simulink model "TosAeb"  (R2021a)
settings: Solver=FixedStepDiscrete  StartTime=0.0  StopTime=inf  FixedStep=0.01  RelTol=1e-3
undefined symbols (not defined in the file or in its folder): ACCModSts, AccSts1, AEB_ObjectMovingOrientation, AEB_ObjectMovingState, CN_BTCActive, CN_CurrentGearR, CN_CurrentGearR_DHT, CN_PTCActive, CN_VDCActive, CN_VSC_EspOff, CN_VSC_FCW_AEB_Error, CN_VSC_FCW_AEB_NoDispay, CN_VSC_FCW_AEB_PerfmDgdtn, CN_VSC_FCW_AEB_TempUnAvlbl, CN_VSC_VehStandstill, CNaccelAvoidMax, CNaccelAvoidMin, CNbrakePedalPress, CNcomfortStrLeftAccelMax, CNcomfortStrLeftAccelMin, CNcomfortStrRightAccelMax, CNcomfortStrRightAccelMin, CNDrvDoorOpen, CNDrvModeEco, CNDrvModeNorm, CNDrvModeOther, CNDrvModeSport, CNDrvSBRreminder, CNNull, CNobjInvalidID, CNPassDoorOpen, CNPassSBRreminder, CNPEBSAccelNegInf, CNPEBSAccelNull, CNPEBSTimeInf, CNPEBSTimeNull, CNSTOPMAX, CNSTOPNULL, CNsubjWidth, CNSwtOFF, CNSwtON, CNTTCMAX, CNTTCNULL, CNVehConfigAEBCycNone, CNVehConfigAEBNone, CNVehConfigAEBPedNone, CNVehConfigFCWNone, CNVehConfigJANone, CollisionSeverity, FALSE, FCWSnvty, JACycObjCalcInfoDefaultParams, JAPedObjCalcInfoDefaultParams, JAVehObjCalcInfoDefaultParams, latCycObjCalcInfoDefaultParams, latPedObjCalcInfoDefaultParams, longObjCalcInfoDefaultParams, ModSts, movingStatus, ObjectType ... (+1112)
libraries: simulink x3031, AEBSFunctionLib x50, Ta_Library x33, AEBSStateMachineLib x23, Mylibrary x12, simulink_extras x7, ObjSelect_lib x3, dspstat3 x2
(part 100 of 102)

== stateflow chart TosAeb/FcwAebJa_Step_sys/ALG_AEBSystem/ALG_AEBController/AEB_LatObjInfoCalcApp/LatCycObjCalc/LatCycObjCalc/latCycObjInfoCalc/ObjColiisionRiskProbCalc/CollisionRiskProbCalc/ObjReginCorrCalc/ObjReginCorrCalc/Chart ==
  note: ObjUp2Dwn
  note: ObjDwn2Up
  note: ObjLeft2Right
  note: ObjRight2Left
  (default) -> (junction 2)
  (junction 2) -> (junction 3) [order 1] : [vxAbsObj < 0]
  (junction 2) -> (junction 26) [order 2]
  (junction 3) -> (junction 5) : {
      | 	tObjStop = abs(vxAbsObj/ax_Max);
      | }
  (junction 26) -> (junction 28) [order 1] : [vxAbsObj > 0]
  (junction 26) -> (junction 30) [order 2] : {
      | 	temp_tPredTimeMax_X = 0;
      | 	temp_tPredTimeMin_X = 0;
      | }
  (junction 28) -> (junction 15) : {
      | 	tObjStop = abs(vxAbsObj/ax_Min);
      | }
  (junction 5) -> (junction 7) [order 1] : [tObjStop < tPredTime]
  (junction 5) -> (junction 11) [order 2] : {
      | 	temp_tPredTimeMax_X = tPredTime;
      | 	temp_tPredTimeMin_X = tPredTime;
      | }
  (junction 7) -> (junction 9) : {
      | 	temp_tPredTimeMax_X = tObjStop;
      | 	temp_tPredTimeMin_X = tPredTime;
      | }
  (junction 15) -> (junction 19) [order 1] : [tObjStop < tPredTime]
  (junction 15) -> (junction 23) [order 2] : {
      | 	temp_tPredTimeMax_X = tPredTime;
      | 	temp_tPredTimeMin_X = tPredTime;
      | }
  (junction 19) -> (junction 21) : {
      | 	temp_tPredTimeMax_X = tPredTime;
      | 	temp_tPredTimeMin_X = tObjStop;
      | }
  (junction 23) -> (junction 30)
  (junction 21) -> (junction 23)
  (junction 11) -> (junction 21)
  (junction 9) -> (junction 11)
  (junction 30) -> (junction 53)
  (junction 53) -> (junction 65) [order 1] : [vyAbsObj < 0]
  (junction 53) -> (junction 36) [order 2]
  (junction 65) -> (junction 64) : {
      | 	tObjStop = abs(vyAbsObj/ay_Max);
      | }
  (junction 36) -> (junction 40) [order 1] : [vyAbsObj > 0]
  (junction 36) -> (junction 35) [order 2] : {
      | 	temp_tPredTimeMax_Y= 0;
      | 	temp_tPredTimeMin_Y= 0;
      | }
  (junction 40) -> (junction 50) : {
      | 	tObjStop = abs(vyAbsObj/ay_Min);
      | }
  (junction 64) -> (junction 56) [order 1] : [tObjStop < tPredTime]
  (junction 64) -> (junction 51) [order 2] : {
      | 	temp_tPredTimeMax_Y = tPredTime;
      | 	temp_tPredTimeMin_Y= tPredTime;
      | }
  (junction 56) -> (junction 42) : {
      | 	temp_tPredTimeMax_Y = tObjStop;
      | 	temp_tPredTimeMin_Y = tPredTime;
      | }
  (junction 50) -> (junction 54) [order 1] : [tObjStop < tPredTime]
  (junction 50) -> (junction 58) [order 2] : {
      | 	temp_tPredTimeMax_Y = tPredTime;
      | 	temp_tPredTimeMin_Y= tPredTime;
      | }
  (junction 54) -> (junction 39) : {
      | 	temp_tPredTimeMax_Y = tPredTime;
      | 	temp_tPredTimeMin_Y = tObjStop;
      | }
  (junction 58) -> (junction 35)
  (junction 39) -> (junction 58)
  (junction 51) -> (junction 39)
  (junction 42) -> (junction 51)
  (junction 35) -> (junction 67) : {
      | 	tPredTimeMax_X = temp_tPredTimeMax_X;
      | 	tPredTimeMin_X = temp_tPredTimeMin_X;
      | 	tPredTimeMax_Y = temp_tPredTimeMax_Y;
      | 	tPredTimeMin_Y = temp_tPredTimeMin_Y;
      | }
  data: ax_Max input; ax_Min input; ay_Max input; ay_Min input; tPredTime input; vxAbsObj input; vyAbsObj input; tPredTimeMax_X output single; tPredTimeMin_X output single; tPredTimeMax_Y output single; tPredTimeMin_Y output single; tObjStop local single; temp_tPredTimeMax_X local single; temp_tPredTimeMax_Y local single; temp_tPredTimeMin_X local single; temp_tPredTimeMin_Y local single

== stateflow chart TosAeb/FcwAebJa_Step_sys/ALG_AEBSystem/ALG_AEBController/AEB_LongTgtInfoCalc/LongObjCalcInfoCalc/ObjAbsInfoTrans/ObjFusQualityCal ==
  state [deltaDxThres,deltaDyThres,deltaFC_FRVxThres,deltaFR_FusVxThres,deltaFC_FusVxThres] = SensorThres(subjVel) [function]
  (default) -> (junction 9)
  (junction 9) -> (junction 11) [order 1] : [ObjectID ~= CNobjInvalidID &&...
      | ObjectTypeCameraConfirmed == true]
  (junction 9) -> (junction 27) [order 2] : {
      |    ObjFusQuality = false;
      | }
  (junction 11) -> (junction 13)
  (junction 13) -> (junction 15) [order 1] : [FR_ObjectID ~= CNobjInvalidID &&...
      | FC_ObjectID ~= CNobjInvalidID]
  (junction 13) -> (junction 24) [order 2] : {
      |    ObjFusQuality = false;
      | }
  (junction 15) -> (junction 17) : {
      |         temp_Dx = abs(FR_Dx - FC_Dx);
      |         temp_Dy = abs(FR_Dy - FC_Dy);
      |         temp_FC_FR_Vx = abs(FC_Vx - FR_Vx);
      |         temp_FC_Fus_Vx = abs(FC_Vx - Fus_Vx);
      |         temp_FR_Fus_Vx = abs(FR_Vx - Fus_Vx);
      |         [deltaDxThres,...
      |          deltaDyThres,...
      |          deltaFC_FRVxThres,...
      |          deltaFR_FusVxThres,...
      |          deltaFC_FusVxThres] = SensorThres(subjVel);
      | }
  (junction 17) -> (junction 19) [order 1] : [temp_Dx > deltaDxThres ||...
      | temp_Dy > deltaDyThres]
  (junction 17) -> (junction 59) [order 2]
  (junction 19) -> (junction 21) : {
      |    ObjFusQuality = false;
      | }
  (junction 59) -> (junction 66) [order 1] : [Fus_ObjectType ==ObjectTypeCopy.CAR ||...
      | Fus_ObjectType ==ObjectTypeCopy.TRUCK]
  (junction 59) -> (junction 33) [order 2] : {
      |    ObjFusQuality = true;
      | }
  (junction 66) -> (junction 68)
  (junction 68) -> (junction 61) [order 1] : [(temp_FC_FR_Vx>deltaFC_FRVxThres&&...
      | temp_FR_Fus_Vx<deltaFR_FusVxThres)||...
      | (temp_FC_FR_Vx<deltaFC_FRVxThres&&...
      | temp_FR_Fus_Vx>deltaFR_FusVxThres)]
  (junction 68) -> (junction 70) [order 2] : {
      |    ObjFusQuality = true;
      | }
  (junction 61) -> (junction 63) : {
      |    ObjFusQuality = false;
      | }
  (junction 24) -> (junction 27)
  (junction 33) -> (junction 24)
  (junction 70) -> (junction 33)
  (junction 63) -> (junction 70)
  (junction 21) -> (junction 63)
  (junction 27) -> (junction 30)
  data: subjVel function_input; deltaDxThres function_output single; deltaDyThres function_output single; deltaFC_FRVxThres function_output; deltaFR_FusVxThres function_output; deltaFC_FusVxThres function_output; ObjectID input size=1; ObjectTypeCameraConfirmed input size=1; Fus_ObjectType input Enum: ObjectTypeCopy; FC_ObjectID input size=1; FR_ObjectID input size=1; FR_Dx input size=1; FR_Dy input size=1; FR_Vx input; FC_Dx input size=1; FC_Dy input size=1; CNobjInvalidID parameter size=1; ObjFusQuality output size=1; temp_Dx local single size=1; temp_Dy local single size=1; deltaDyThres local single size=1 =single(0); deltaDxThres local single size=1 =single(0); temp_FC_Fus_Vx local single; deltaFC_FRVxThres local single =single(0); deltaFR_FusVxThres local single =single(0); FC_Vx input; Fus_Vx input; subjVel input size=1; temp_FC_FR_Vx local single; temp_FR_Fus_Vx local single; deltaFC_FusVxThres local single =single(0)

== stateflow chart TosAeb/FcwAebJa_Step_sys/ALG_AEBSystem/ALG_AEBController/AEB_LatObjInfoCalcApp/LatCycObjCalc/LatCycObjCalc/latCycObjInfoCalc/ObjColiisionRiskProbCalc/CollisionRiskProbCalcFlow ==
  (default) -> (junction 11)
  (junction 11) -> (junction 2) : {
      | 	tPredictTime = 0;
      | 	temp_collisionRiskProbMax = 0;
      | }
  (junction 2) -> (junction 6) [order 1] : [tPredictTime < tMaxPredTime]
  (junction 41) -> (junction 2)
  (junction 2) -> (junction 13) [order 2] : {
      | 	collisionRiskProbMax = temp_collisionRiskProbMax;
      | 	preCollisonMaxTime = temp_preCollisonMaxTime;
      | 	dxCollisionRiskProbOut = dxCollisionRiskProb;
      | 	dyCollisionRiskProbOut = dyCollisionRiskProb;
      | }
  (junction 6) -> (junction 32) : {
      | 	tPredictTimeOut = tPredictTime;
      | 	CollisionRiskProbCalcFunc;
      | }
  (junction 32) -> (junction 34) [order 1] : [collisionRiskProb > temp_collisionRiskProbMax]
  (junction 32) -> (junction 8) [order 2]
  (junction 34) -> (junction 35) : {
      | 	temp_collisionRiskProbMax = collisionRiskProb;
      | 	temp_preCollisonMaxTime =  tPredictTime;
      | }
  (junction 35) -> (junction 8)
  (junction 8) -> (junction 41) : {
      | 	tPredictTime += stepTime;
      | }
  data: collisionRiskProbMax output single; dxCollisionRiskProbOut output single; dyCollisionRiskProbOut output single; preCollisonMaxTime output single; stepTime input single; tMaxPredTime input single; collisionRiskProb input single; dyCollisionRiskProb input single; tPredictTimeOut output single; tPredictTime local single; temp_collisionRiskProbMax local single; temp_preCollisonMaxTime local single; dxCollisionRiskProb input single
  events: CollisionRiskProbCalcFunc output FUNCTION_CALL_EVENT

== stateflow chart TosAeb/FcwAebJa_Step_sys/ALG_AEBSystem/ALG_AEBController/AEB_LatObjInfoCalcApp/LatCycObjCalc/LatCycObjCalc/latCycObjInfoCalc/ObjCrossFlgCal/CollisionProbCalcFlow ==
  (default) -> (junction 11)
  (junction 11) -> (junction 2) : {
      |   tPredictTime = 0;
      |   TTC = 256;
      | }
  (junction 2) -> (junction 6) [order 1] : [tPredictTime < tMaxPredTime]
  (junction 30) -> (junction 2) [order 2]
  (junction 2) -> (junction 13) [order 2]
  (junction 6) -> (junction 8) : {
      | 	tPredictTimeOut = tPredictTime;
      | 	CollisionProbFunc;
      | }
  (junction 8) -> (junction 30) : {
      | 	tPredictTime += stepTime;
      | }
  (junction 30) -> (junction 22) [order 1] : [preCollisionProb == true]
  (junction 22) -> (junction 13) : {
      |   TTC = tPredictTimeOut;
      | }
  (junction 13) -> (junction 25)
  data: stepTime input single; tMaxPredTime input single; tPredictTimeOut output single; tPredictTime local single; preCollisionProb input; TTC output single
  events: CollisionProbFunc output FUNCTION_CALL_EVENT

== stateflow chart TosAeb/FcwAebJa_Step_sys/ALG_AEBSystem/ALG_AEBController/AEB_LatObjInfoCalcApp/LatCycObjCalc/LatCycObjCalc/latCycObjInfoCalc/ObjCrossFlgCal/CycPreCollisionCalcFunc/ObjLenthWidthCalc/ObjLengthWidthCalc ==
  (default) -> (junction 10) : {
      | 	ObjLengthCorr = P_LatCycCA_PreCollisionCycLengthThres;
      | 	ObjWidthCorr = P_LatCycCA_PreCollisionCycWidthThres;
      | }
  (junction 10) -> (junction 12) [order 1] : [(abs(ObjectYawAngle) > 45 &&...
      | abs(ObjectYawAngle) < 135)||...
      | ObjectMovingOrientation == AEB_ObjectMovingOrientation.Crossing]
  (junction 10) -> (junction 16) [order 2]
  (junction 12) -> (junction 14) : {
      | 	ObjLengthCorr = ObjectWidth;
      | 	ObjWidthCorr = ObjectLength;
      | }
  (junction 14) -> (junction 16)
  (junction 16) -> (junction 19)
  data: ObjectYawAngle input; ObjectMovingOrientation input; ObjectLength input; ObjectWidth input; ObjLengthCorr output single; ObjWidthCorr output single; P_LatCycCA_PreCollisionCycLengthThres input; P_LatCycCA_PreCollisionCycWidthThres input

== stateflow chart TosAeb/FcwAebJa_Step_sys/ALG_AEBSystem/ALG_AEBController/AEB_LatObjInfoCalcApp/LatCycObjCalc/LatCycObjCalc/latCycObjInfoCalc/ObjCrossFlgCal/CycPreCollisionCalcFunc/ObjLenthWidthCalc/ObjLengthWidthCalc1 ==
  (default) -> (junction 32)
  (junction 32) -> (junction 34) [order 1] : [ObjectTypeCameraConfirmed == true]
  (junction 32) -> (junction 30) [order 2] : {
      | 	ObjectLength = P_LatCycCA_PreCollisionCycLengthThres;
      | 	ObjectWidth = P_LatCycCA_PreCollisionCycWidthThres;
      | }
  (junction 34) -> (junction 36) : {
      | 	ObjectLength = ObjectLengthIn;
      | 	ObjectWidth = ObjectWidthIn;
      | }
  (junction 36) -> (junction 30)
  (junction 30) -> (junction 10)
  (junction 10) -> (junction 12) [order 1] : [(abs(ObjectYawAngle) > 45 &&...
      | abs(ObjectYawAngle) < 135)||...
      | ObjectMovingOrientation == AEB_ObjectMovingOrientation.Crossing]
  (junction 10) -> (junction 16) [order 2]
  (junction 12) -> (junction 14) : {
      |       temp = ObjectLength;
      | 	ObjectLength = ObjectWidth;
      | 	ObjectWidth = temp;
      | }
  (junction 14) -> (junction 16)
  (junction 16) -> (junction 19) : {
      |    ObjectLength = min(ObjectLength,2.5);
      |    ObjectWidth = min(ObjectWidth,1.5);
      | }
  data: ObjectTypeCameraConfirmed input boolean; ObjectLengthIn input; ObjectWidthIn input; ObjectYawAngle input; ObjectMovingOrientation input; ObjectLength output single; ObjectWidth output single; P_LatCycCA_PreCollisionCycLengthThres input; P_LatCycCA_PreCollisionCycWidthThres input; temp local single

== stateflow chart TosAeb/FcwAebJa_Step_sys/ALG_AEBSystem/ALG_AEBController/AEB_LatObjInfoCalcApp/LatCycObjCalc/LatCycObjCalc/latCycObjInfoCalc/ObjCrossFlgCal/CycPreCollisionCalcFunc/PreCrossFlgCalcFlow ==
  state pedPreCollisionFlg = VectorCrossAlgorithm(x1,y1,x2,y2,m1,n1,m2,n2) [function]
  (default) -> (junction 59) : {
      | 	pedPreCollisionFlg = false;
      | 	i = 0;
      | }
  (junction 59) -> (junction 58) [order 1] : [ i < 4]
  (junction 58) -> (junction 48) : {
      | 	p = (i+1)%%4;
      | 	j = 0;
      | }
  (junction 48) -> (junction 46) [order 1] : [j < 4]
  (junction 46) -> (junction 49) : {
      | 	q = (j+1)%%4;
      | 	pedPreCollisionFlg = VectorCrossAlgorithm(subjDxPre[i],subjDyPre[i],subjDxPre[p],subjDyPre[p],...
      | 	objDxPre[j],objDyPre[j],objDxPre[q],objDyPre[q]);
      | }
  (junction 60) -> (junction 59) : {
      | 	i++;
      | }
  (junction 59) -> (junction 61) [order 2]
  (junction 49) -> (junction 48) [order 2] : {
      | 	j++;
      | }
  (junction 48) -> (junction 60) [order 2]
  (junction 49) -> (junction 64) [order 1] : [pedPreCollisionFlg == true]
  (junction 64) -> (junction 61)
  (junction 61) -> (junction 67)
  data: x1 function_input single; y1 function_input single; x2 function_input single; y2 function_input single; m1 function_input single; n1 function_input single; m2 function_input single; n2 function_input single; pedPreCollisionFlg function_output; subjDxPre input single; subjDyPre input single; objDxPre input single; objDyPre input single; j local single; i local single; p local single; q local single; pedPreCollisionFlg output boolean

== stateflow chart TosAeb/FcwAebJa_Step_sys/ALG_AEBSystem/ALG_AEBController/AEB_LatObjInfoCalcApp/LatCycObjCalc/TargetSelectCalc/tgtObjFlow1 ==
  state TargetSelectFunc [function]
    (default) -> (junction 63)
    (junction 63) -> (junction 75) [order 1] : [ObjPreSelectCondFufilled(index) == false]
    (junction 63) -> (junction 94) [order 2] : {temp = latCycCalcInfo(index).CollisionRiskProb;}
    (junction 75) -> (junction 72)
    (junction 94) -> (junction 93) [order 1] : %[latCycCalcInfo(index).CollisionRiskProb > 0.55]
        | [temp > P_LatCycCA_SelectObjCollisionRiskProbThres]
    (junction 94) -> (junction 187) [order 2]
    (junction 93) -> (junction 225) : {
        | 	tgtIsFind = true;
        | }
    (junction 225) -> (junction 227) [order 1] : [ObjPreSelectCondFufilled(tgtIndex) == false]
    (junction 225) -> (junction 185) [order 2]
    (junction 227) -> (junction 229) : {
        | 	tgtIndex = index;
        | }
    (junction 185) -> (junction 143) [order 1] : [latCycCalcInfo(index).CollisionRiskProb > ...
        | latCycCalcInfo(tgtIndex).CollisionRiskProb]
    (junction 185) -> (junction 149) [order 2]
    (junction 143) -> (junction 145) : {
        | 	tgtIndex = index;
        | }
    (junction 149) -> (junction 151) [order 1] : [abs(latCycCalcInfo(index).CollisionRiskProb) == ...
        | abs(latCycCalcInfo(tgtIndex).CollisionRiskProb)]
    (junction 149) -> (junction 69) [order 2]
    (junction 151) -> (junction 157) : {
        | 	temp1=abs(latCycCalcInfo(index).latCycObjRawInfo.dy);
        | 	temp2 = abs(latCycCalcInfo(tgtIndex).latCycObjRawInfo.dy);
        | }
    (junction 157) -> (junction 158) [order 1] : [temp1 <= temp2]
    (junction 157) -> (junction 153) [order 2]
    (junction 158) -> (junction 160) : {
        | 	tgtIndex = index;
        | }
    (junction 229) -> (junction 145)
    (junction 72) -> (junction 229)
    (junction 145) -> (junction 160)
    (junction 69) -> (junction 187)
    (junction 153) -> (junction 69)
    (junction 160) -> (junction 153)
    (junction 187) -> (junction 81)
  state TgtIndexSearch [function]
    (default) -> (junction 233)
    (junction 233) -> (junction 234) : {
        |     index = 1;
        | }
    (junction 234) -> (junction 235) [order 1] : [index <= 10]
    (junction 238) -> (junction 234)
    (junction 234) -> (junction 239) [order 2]
    (junction 235) -> (junction 236)
    (junction 236) -> (junction 237) [order 1] : [latCycCalcInfo(index).latCycObjRawInfo.ObjectID == tgtIndexK1]
    (junction 236) -> (junction 238) [order 2] : {
        |    index = index+1;
        | }
    (junction 237) -> (junction 240) : {
        |    tgtIndexK1 = index;
        | }
    (junction 240) -> (junction 239)
    (junction 239) -> (junction 241)
  (default) -> (junction 191) : {
      | 	tgtIndex = 1;
      | 	tgtIsFind = false;
      |       tgtIndexK1 = uint8(1);
      | }
  (junction 191) -> (junction 198)
  (junction 198) -> (junction 196) [order 1] : [TargetIDK1 ~= 0]
  (junction 198) -> (junction 216) [order 2]
  (junction 196) -> (junction 200) : {
      |    TgtIndexSearch();
      | }
  (junction 200) -> (junction 193) [order 1] : [ObjPreSelectCondFufilled(tgtIndexK1) == true]
  (junction 200) -> (junction 213) [order 2]
  (junction 193) -> (junction 205)
  (junction 213) -> (junction 216)
  (junction 205) -> (junction 213) [order 2]
  (junction 205) -> (junction 207) [order 1] : [latCycCalcInfo(tgtIndexK1).CollisionRiskProb >P_LatCycCA_SelectTgtCollisionRiskProbThres ]
  (junction 216) -> (junction 112) : {
      | 	index = uint8(1);
      | }
  (junction 207) -> (junction 211) : {
      | 	tgtIndex = tgtIndexK1;
      | 	tgtIsFind = true;
      | }
  (junction 112) -> (junction 110) [order 1] : [index <= 10]
  (junction 169) -> (junction 112)
  (junction 112) -> (junction 209) [order 2]
  (junction 110) -> (junction 113) : {
      | 	TargetSelectFunc();
      | }
  (junction 113) -> (junction 169) : {
      | 	index = index + 1;
      | }
  (junction 211) -> (junction 209)
  (junction 209) -> (junction 114)
  (junction 114) -> (junction 172) [order 1] : [tgtIsFind == true]
  (junction 114) -> (junction 176) [order 2] : {
      | 	tgtObj = latCycObjCalcInfoDefaultParams;
      | }
  (junction 172) -> (junction 174) : {
      | 	tgtObj = latCycCalcInfo(tgtIndex);
      | }
  (junction 174) -> (junction 176)
  (junction 176) -> (junction 179)
  data: latCycCalcInfo input Bus: BusLatCycObjCalcInfo size=10; ObjPreSelectCondFufilled input size=10; index local uint8; tgtObj output Bus: BusLatCycObjCalcInfo; temp1 local; temp2 local; tgtIsFind output; latCycObjCalcInfoDefaultParams parameter Bus: BusLatCycObjCalcInfo; tgtIndex output uint8; temp local; P_LatCycCA_SelectObjCollisionRiskProbThres input; P_LatCycCA_SelectTgtCollisionRiskProbThres input; TargetIDK1 input uint16; tgtIndexK1 local uint8

== stateflow chart TosAeb/FcwAebJa_Step_sys/ALG_AEBSystem/ALG_AEBController/AEB_LatObjInfoCalcApp/LatPedObjCalc/LatPedCollisonAssessInfo/LatPedCollisonAssessInfo/LatPedCollisionSeverityCalc/LatCollsionSeverityJudgeFlow ==
  note: DistanceTriggerForPrefill == true
  note: 变更1和2的条件
  (default) -> (junction 88)
  (junction 88) -> (junction 89) [order 1] : [ latCollsionSeverityK1== CollisionSeverity.None]
  (junction 88) -> (junction 92) [order 2]
  (junction 89) -> (junction 90) : {
      | 	PseverityLowThres = ...
      | P_LatPedCA_Lvl1SeverityThresCurrentLvl0;
      | 	PseverityMediumThres = ...
      | P_LatPedCA_Lvl2SeverityThresCurrentLvl0;
      | 	PseverityHighThres = ...
      | P_LatPedCA_Lvl3SeverityThresCurrentLvl0;
      | }
  (junction 92) -> (junction 93) [order 1] : [ latCollsionSeverityK1== CollisionSeverity.Low]
  (junction 92) -> (junction 96) [order 2]
  (junction 93) -> (junction 94) : {
      | 	PseverityLowThres = ...
      | P_LatPedCA_Lvl1SeverityThresCurrentLvl1;
      | 	PseverityMediumThres = ...
      | P_LatPedCA_Lvl2SeverityThresCurrentLvl1;
      | 	PseverityHighThres = ...
      | P_LatPedCA_Lvl3SeverityThresCurrentLvl1;
      | }
  (junction 96) -> (junction 97) [order 1] : [ latCollsionSeverityK1== CollisionSeverity.Medium]
  (junction 96) -> (junction 100) [order 2] : {
      | 	PseverityLowThres = ...
      | P_LatPedCA_Lvl1SeverityThresCurrentLvl3;
      | 	PseverityMediumThres = ...
      | P_LatPedCA_Lvl2SeverityThresCurrentLvl3;
      | 	PseverityHighThres = ...
      | P_LatPedCA_Lvl3SeverityThresCurrentLvl3;
      | }
  (junction 97) -> (junction 98) : {
      | 	PseverityLowThres = ...
      | P_LatPedCA_Lvl1SeverityThresCurrentLvl2;
      | 	PseverityMediumThres = ...
      | P_LatPedCA_Lvl2SeverityThresCurrentLvl2;
      | 	PseverityHighThres = ...
      | P_LatPedCA_Lvl3SeverityThresCurrentLvl2;
      | }
  (junction 98) -> (junction 100)
  (junction 94) -> (junction 98)
  (junction 90) -> (junction 94)
  (junction 100) -> (junction 106)
  (junction 106) -> (junction 108) [order 1] : [TargetID == CNobjInvalidID]
  (junction 108) -> (junction 144) : {
      | 	tempLatCollsionSeverity = CollisionSeverity.None;
      | }
  (junction 106) -> (junction 124) [order 2]
  (junction 124) -> (junction 120) [order 1] : [ pedColliisonRisk > PseverityHighThres]
  (junction 124) -> (junction 189) [order 2]
  (junction 120) -> (junction 155)
  (junction 155) -> (junction 189) [order 2]
  (junction 155) -> (junction 156) [order 1] : [ longDistCond4CollisionSeverityHigh == true]
  (junction 189) -> (junction 187)
  (junction 156) -> (junction 158) : {
      | 	tempLatCollsionSeverity = ...
      | 	CollisionSeverity.High;
      | }
  (junction 187) -> (junction 113) [order 1] : [ pedColliisonRisk > PseverityMediumThres]
  (junction 187) -> (junction 193) [order 2]
  (junction 113) -> (junction 163)
  (junction 163) -> (junction 193) [order 2]
  (junction 163) -> (junction 164) [order 1] : [longDistCond4CollisionSeverityMedium == true]
  (junction 193) -> (junction 196)
  (junction 164) -> (junction 112) : {
      | 	tempLatCollsionSeverity = ...
      | 	CollisionSeverity.Medium;
      | }
  (junction 196) -> (junction 136) [order 1] : [ pedColliisonRisk > PseverityLowThres]
  (junction 196) -> (junction 198) [order 2]
  (junction 136) -> (junction 171)
  (junction 171) -> (junction 198) [order 2]
  (junction 171) -> (junction 172) [order 1] : [longDistCond4CollisionSeverityLow == true]
  (junction 198) -> (junction 119) : {
      | 	tempLatCollsionSeverity = CollisionSeverity.None;
      | }
  (junction 172) -> (junction 174) : {
      | 	tempLatCollsionSeverity = ...
      | 	CollisionSeverity.Low;
      | }
  (junction 158) -> (junction 112)
  (junction 144) -> (junction 158)
  (junction 112) -> (junction 174)
  (junction 174) -> (junction 119)
  (junction 119) -> (junction 132) : {
      | 	latCollsionSeverity = tempLatCollsionSeverity;
      | }
  data: TargetID input; pedColliisonRisk input single; longDistCond4CollisionSeverityLow input; longDistCond4CollisionSeverityMedium input; longDistCond4CollisionSeverityHigh input; latCollsionSeverity output Enum: CollisionSeverity; CNobjInvalidID parameter; PseverityLowThres local single; PseverityMediumThres local single; PseverityHighThres local single; tempLatCollsionSeverity local Enum: CollisionSeverity; P_LatPedCA_Lvl1SeverityThresCurrentLvl0 input; P_LatPedCA_Lvl2SeverityThresCurrentLvl0 input; P_LatPedCA_Lvl3SeverityThresCurrentLvl0 input; P_LatPedCA_Lvl1SeverityThresCurrentLvl1 input; P_LatPedCA_Lvl2SeverityThresCurrentLvl1 input; P_LatPedCA_Lvl3SeverityThresCurrentLvl1 input; P_LatPedCA_Lvl1SeverityThresCurrentLvl2 input; P_LatPedCA_Lvl2SeverityThresCurrentLvl2 input; P_LatPedCA_Lvl3SeverityThresCurrentLvl2 input; P_LatPedCA_Lvl1SeverityThresCurrentLvl3 input; P_LatPedCA_Lvl2SeverityThresCurrentLvl3 input; P_LatPedCA_Lvl3SeverityThresCurrentLvl3 input; latCollsionSeverityK1 input Enum: CollisionSeverity

== stateflow chart TosAeb/FcwAebJa_Step_sys/ALG_AEBSystem/ALG_AEBController/AEB_LatObjInfoCalcApp/LatPedObjCalc/LatPedObjCalc/latPedObjInfoCalc/ObjColiisionRiskProbCalc/CollisionRiskProbCalc/CollisionRiskProbCalc/dxOverlapFlow ==
  (default) -> (junction 32) : {
      | 	dxOverlapLimit = dxReginObjRUCorr - dxReginObjLLCorr;
      | 	dxOverlapLimit = max(dxOverlapLimit,0.1);
      | }
  (junction 32) -> (junction 34) [order 1] : [dxReginObjLLCorr > dxReginEgoRUCorr || ...
      | dxReginObjRUCorr < dxReginEgoLLCorr]
  (junction 32) -> (junction 2) [order 2]
  (junction 34) -> (junction 36) : {
      | 	dxOverlap = 0;
      | }
  (junction 2) -> (junction 3) [order 1] : [dxReginEgoRUCorr - dxReginEgoLLCorr < dxOverlapLimit && ...
      | dxReginEgoLLCorr >= dxReginObjLLCorr &&...
      | dxReginEgoRUCorr < dxReginObjRUCorr]
  (junction 2) -> (junction 7) [order 2]
  (junction 3) -> (junction 5) : {
      | 	dxOverlap = dxReginEgoRUCorr - dxReginEgoLLCorr;
      | }
  (junction 7) -> (junction 9) [order 1] : [dxReginEgoRUCorr - dxReginObjLLCorr < dxOverlapLimit &&...
      | dxReginEgoRUCorr - dxReginObjLLCorr > 0 && ...
      | dxReginEgoLLCorr < dxReginObjLLCorr]
  (junction 7) -> (junction 13) [order 2]
  (junction 9) -> (junction 11) : {
      | 	dxOverlap = dxReginEgoRUCorr - dxReginObjLLCorr;
      | }
  (junction 13) -> (junction 15) [order 1] : [dxReginObjRUCorr - dxReginEgoLLCorr< dxOverlapLimit && ...
      | dxReginObjRUCorr - dxReginEgoLLCorr> 0 && ...
      | dxReginEgoRUCorr > dxReginObjRUCorr]
  (junction 13) -> (junction 19) [order 2] : {
      | 	dxOverlap= dxOverlapLimit;
      | }
  (junction 15) -> (junction 17) : {
      | 	dxOverlap = dxReginObjRUCorr - dxReginEgoLLCorr;
      | }
  (junction 17) -> (junction 19)
  (junction 11) -> (junction 17)
  (junction 5) -> (junction 11)
  (junction 36) -> (junction 5)
  (junction 19) -> (junction 22)
  data: dxReginEgoRUCorr input single; dxReginEgoLLCorr input single; dxReginObjRUCorr input single; dxReginObjLLCorr input single; dxOverlap output single; dxOverlapLimit output single

== stateflow chart TosAeb/FcwAebJa_Step_sys/ALG_AEBSystem/ALG_AEBController/AEB_LatObjInfoCalcApp/LatPedObjCalc/LatPedObjCalc/latPedObjInfoCalc/ObjColiisionRiskProbCalc/CollisionRiskProbCalc/CollisionRiskProbCalc/dyOverlapFlow ==
  (default) -> (junction 32) : {
      | 	dyOverlapLimit = dyReginObjLLCorr- dyReginObjRUCorr;
      | 	dyOverlapLimit = max(dyOverlapLimit,0.1);
      | }
  (junction 32) -> (junction 34) [order 1] : [dyReginObjLLCorr < dyReginEgoRUCorr || ...
      | dyReginObjRUCorr > dyReginEgoLLCorr]
  (junction 32) -> (junction 2) [order 2]
  (junction 34) -> (junction 36) : {
      | 	dyOverlap = 0;
      | }
  (junction 2) -> (junction 3) [order 1] : [dyReginEgoLLCorr - dyReginEgoRUCorr < dyOverlapLimit && ...
      | dyReginEgoLLCorr <= dyReginObjLLCorr &&...
      | dyReginEgoRUCorr >= dyReginObjRUCorr]
  (junction 2) -> (junction 7) [order 2]
  (junction 3) -> (junction 5) : {
      | 	dyOverlap = dyReginEgoLLCorr - dyReginEgoRUCorr;
      | }
  (junction 7) -> (junction 9) [order 1] : [dyReginObjLLCorr - dyReginEgoRUCorr < dyOverlapLimit &&...
      | dyReginObjLLCorr - dyReginEgoRUCorr >0 && ...
      | dyReginEgoLLCorr > dyReginObjLLCorr]
  (junction 7) -> (junction 13) [order 2]
  (junction 9) -> (junction 11) : {
      | 	dyOverlap = dyReginObjLLCorr - dyReginEgoRUCorr;
      | }
  (junction 13) -> (junction 15) [order 1] : [dyReginEgoLLCorr - dyReginObjRUCorr < dyOverlapLimit && ...
      | dyReginEgoLLCorr - dyReginObjRUCorr > 0 && ...
      | dyReginEgoRUCorr < dyReginObjRUCorr]
  (junction 13) -> (junction 19) [order 2] : {
      | 	dyOverlap= dyOverlapLimit;
      | }
  (junction 15) -> (junction 17) : {
      | 	dyOverlap = dyReginEgoLLCorr - dyReginObjRUCorr;
      | }
  (junction 17) -> (junction 19)
  (junction 11) -> (junction 17)
  (junction 5) -> (junction 11)
  (junction 36) -> (junction 5)
  (junction 19) -> (junction 22)
  data: dyReginEgoRUCorr input single; dyReginEgoLLCorr input single; dyReginObjRUCorr input single; dyReginObjLLCorr input single; dyOverlap output single; dyOverlapLimit output single

== matlab function TosAeb/FcwAebJa_Step_sys/ALG_AEBSystem/ALG_AEBController/AEB_LatObjInfoCalcApp/LatPedObjCalc/LatPedObjCalc/latPedObjInfoCalc/ObjColiisionRiskProbCalc/CollisionRiskProbCalc/MATLAB Function ==
  data: dxReginEgoRUCorr input; dyReginEgoRUCorr input; dyReginEgoLLCorr input; dxReginEgoLLCorr input; dxReginObjRUCorr input; dyReginObjRUCorr input; dxReginObjLLCorr input; dyReginObjLLCorr input; collisionRiskProb input; dyCollisionRiskProb input; dxCollisionRiskProb input
function OverlapPlot(dxReginEgoRUCorr, dyReginEgoRUCorr, dyReginEgoLLCorr, dxReginEgoLLCorr,...
    dxReginObjRUCorr, dyReginObjRUCorr, dxReginObjLLCorr, dyReginObjLLCorr,...
    collisionRiskProb, dyCollisionRiskProb, dxCollisionRiskProb)
coder.extrinsic('plot')
coder.extrinsic('num2str')
dxReginEgoLUCorr = dxReginEgoRUCorr;
dyReginEgoLUCorr = dyReginEgoLLCorr;
dxReginEgoRLCorr = dxReginEgoLLCorr;
dyReginEgoRLCorr = dyReginEgoRUCorr;

dxReginObjLUCorr = dxReginObjRUCorr;
dyReginObjLUCorr = dyReginObjLLCorr;
dxReginObjRLCorr = dxReginObjLLCorr;
dyReginObjRLCorr = dyReginObjRUCorr;

xEgo = [dxReginEgoRUCorr,dxReginEgoLUCorr,dxReginEgoLLCorr,dxReginEgoRLCorr];
yEgo = [dyReginEgoRUCorr,dyReginEgoLUCorr,dyReginEgoLLCorr,dyReginEgoRLCorr];
xObj = [dxReginObjRUCorr,dxReginObjLUCorr,dxReginObjLLCorr,dxReginObjRLCorr];
yObj = [dyReginObjRUCorr,dyReginObjLUCorr,dyReginObjLLCorr,dyReginObjRLCorr];

xEgo1 = yEgo;
yEgo1 = -xEgo;
xObj1 = yObj;
yObj1 = -xObj;

plot(xEgo1,yEgo1,'-o',...
    'LineWidth',1','FaceColor','red','EdgeColor','red','FaceAlpha',.2)
hold on
plot(xObj1,yObj1,'-o',...
    'LineWidth',1','FaceColor','blue','EdgeColor','blue','FaceAlpha',.4)

txtEgoRU = ['(',num2str(xEgo1(1)),',',num2str(yEgo1(1)),')'];
text(xEgo1(1),yEgo1(1),txtEgoRU,'Color','red','FontSize',14)
txtEgoLL = ['(',num2str(xEgo1(3)),',',num2str(yEgo1(3)),')'];
text(xEgo1(1),yEgo1(1),txtEgoLL,'Color','red','FontSize',14)

%     text(sum(xEgo1)/4,sum(xEgo1)/4,['dyOverlap is 1 and dxOverlap is 0'])
%     pause(1);

== stateflow chart TosAeb/FcwAebJa_Step_sys/ALG_AEBSystem/ALG_AEBController/AEB_LatObjInfoCalcApp/LatPedObjCalc/LatPedObjCalc/latPedObjInfoCalc/ObjColiisionRiskProbCalc/CollisionRiskProbCalc/ObjReginCorrCalc/ObjLengthWidthCalc/ObjLengthWidthCalc ==
  (default) -> (junction 10) : {
      | 	ObjLengthCorr = P_LatPedCA_CollisionRiskProbLengthThres;
      | 	ObjWidthCorr = P_LatPedCA_CollisionRiskProbWidthThres;
      | }
  (junction 10) -> (junction 12) [order 1] : [(abs(ObjectYawAngle) > 45 &&...
      | abs(ObjectYawAngle) < 135)||...
      | ObjectMovingOrientation == AEB_ObjectMovingOrientation.Crossing]
  (junction 10) -> (junction 16) [order 2]
  (junction 12) -> (junction 14) : {
      | 	ObjLengthCorr = ObjectWidth;
      | 	ObjWidthCorr = ObjectLength;
      | }
  (junction 14) -> (junction 16)
  (junction 16) -> (junction 19)
  data: ObjectWidth input; ObjectYawAngle input; ObjectMovingOrientation input; ObjectLength input; ObjLengthCorr output single; ObjWidthCorr output single; P_LatPedCA_CollisionRiskProbLengthThres input; P_LatPedCA_CollisionRiskProbWidthThres input

== stateflow chart TosAeb/FcwAebJa_Step_sys/ALG_AEBSystem/ALG_AEBController/AEB_LatObjInfoCalcApp/LatPedObjCalc/LatPedObjCalc/latPedObjInfoCalc/ObjColiisionRiskProbCalc/CollisionRiskProbCalc/ObjReginCorrCalc/ObjLengthWidthCalc/ObjLengthWidthCalc1 ==
  (default) -> (junction 32)
  (junction 32) -> (junction 34) [order 1] : [ObjectTypeCameraConfirmed == true]
  (junction 32) -> (junction 30) [order 2] : {
      | 	ObjectLength = P_LatPedCA_CollisionRiskProbLengthThres;
      | 	ObjectWidth = P_LatPedCA_CollisionRiskProbWidthThres;
      | }
  (junction 34) -> (junction 36) : {
      | 	ObjectLength = ObjectLengthIn;
      | 	ObjectWidth = ObjectWidthIn;
      | }
  (junction 36) -> (junction 30)
  (junction 30) -> (junction 10)
  (junction 10) -> (junction 12) [order 1] : [(abs(ObjectYawAngle) > 45 &&...
      | abs(ObjectYawAngle) < 135)||...
      | ObjectMovingOrientation == AEB_ObjectMovingOrientation.Crossing]
  (junction 10) -> (junction 16) [order 2]
  (junction 12) -> (junction 14) : {
      |       temp = ObjectLength;
      | 	ObjectLength = ObjectWidth;
      | 	ObjectWidth = temp;
      | }
  (junction 14) -> (junction 16)
  (junction 16) -> (junction 19) : {
      |    ObjectLength = min(ObjectLength,1.5);
      |    ObjectWidth = min(ObjectWidth,1.5);
      | }
  data: ObjectTypeCameraConfirmed input boolean; ObjectLengthIn input; ObjectWidthIn input; ObjectYawAngle input; ObjectMovingOrientation input; ObjectLength output single; ObjectWidth output single; P_LatPedCA_CollisionRiskProbLengthThres input; P_LatPedCA_CollisionRiskProbWidthThres input; temp local single

== stateflow chart TosAeb/FcwAebJa_Step_sys/ALG_AEBSystem/ALG_AEBController/AEB_LatObjInfoCalcApp/LatPedObjCalc/LatPedObjCalc/latPedObjInfoCalc/ObjColiisionRiskProbCalc/CollisionRiskProbCalc/ObjReginCorrCalc/PedMaxAccelCalc/PedMovingMode ==
  (default) -> (junction 2)
  (junction 2) -> (junction 3) [order 1] : [vxAbs < P_LatPedCA_CollisionRiskProbPedStopStsVelThres]
  (junction 2) -> (junction 7) [order 2]
  (junction 3) -> (junction 5) : {
      | 	pedMovingState = 0;
      | }
  (junction 7) -> (junction 9) [order 1] : [vxAbs < P_LatPedCA_CollisionRiskProbWalkingStsVelThres]
  (junction 7) -> (junction 14) [order 2]
  (junction 9) -> (junction 11) : {
      | 	pedMovingState = 1;
      | }
  (junction 14) -> (junction 16) [order 1] : [vxAbs < P_LatPedCA_CollisionRiskProbJoggingStsVelThres]
  (junction 14) -> (junction 20) [order 2] : {
      | 	pedMovingState = 3;
      | }
  (junction 16) -> (junction 18) : {
      | 	pedMovingState = 2;
      | }
  (junction 18) -> (junction 20)
  (junction 11) -> (junction 18)
  (junction 5) -> (junction 11)
  (junction 20) -> (junction 24)
  data: vxAbs input; P_LatPedCA_CollisionRiskProbPedStopStsVelThres input; pedMovingState output single; P_LatPedCA_CollisionRiskProbWalkingStsVelThres input; P_LatPedCA_CollisionRiskProbJoggingStsVelThres input

== stateflow chart TosAeb/FcwAebJa_Step_sys/ALG_AEBSystem/ALG_AEBController/AEB_LatObjInfoCalcApp/LatPedObjCalc/LatPedObjCalc/latPedObjInfoCalc/ObjColiisionRiskProbCalc/CollisionRiskProbCalcFlow ==
  (default) -> (junction 11)
  (junction 11) -> (junction 2) : {
      | 	tPredictTime = 0;
      | 	temp_collisionRiskProbMax = 0;
      | }
  (junction 2) -> (junction 6) [order 1] : [tPredictTime < tMaxPredTime]
  (junction 41) -> (junction 2)
  (junction 2) -> (junction 13) [order 2] : {
      | 	collisionRiskProbMax = temp_collisionRiskProbMax;
      | 	preCollisonMaxTime = temp_preCollisonMaxTime;
      | 	dxCollisionRiskProbOut = dxCollisionRiskProb;
      | 	dyCollisionRiskProbOut = dyCollisionRiskProb;
      | }
  (junction 6) -> (junction 32) : {
      | 	tPredictTimeOut = tPredictTime;
      | 	CollisionRiskProbCalcFunc;
      | }
  (junction 32) -> (junction 34) [order 1] : [collisionRiskProb > temp_collisionRiskProbMax]
  (junction 32) -> (junction 8) [order 2]
  (junction 34) -> (junction 35) : {
      | 	temp_collisionRiskProbMax = collisionRiskProb;
      | 	temp_preCollisonMaxTime =  tPredictTime;
      | }
  (junction 35) -> (junction 8)
  (junction 8) -> (junction 41) : {
      | 	tPredictTime += stepTime;
      | }
  data: collisionRiskProbMax output single; dxCollisionRiskProbOut output single; dyCollisionRiskProbOut output single; preCollisonMaxTime output single; stepTime input single; tMaxPredTime input single; collisionRiskProb input single; dyCollisionRiskProb input single; tPredictTimeOut output single; tPredictTime local single; temp_collisionRiskProbMax local single; temp_preCollisonMaxTime local single; dxCollisionRiskProb input single
  events: CollisionRiskProbCalcFunc output FUNCTION_CALL_EVENT

== stateflow chart TosAeb/FcwAebJa_Step_sys/ALG_AEBSystem/ALG_AEBController/AEB_LatObjInfoCalcApp/LatPedObjCalc/LatPedObjCalc/latPedObjInfoCalc/ObjCrossFlgCal/CollisionProbCalcFlow ==
  (default) -> (junction 11)
  (junction 11) -> (junction 2) : {
      |   tPredictTime = 0;
      |   TTC = 256;
      | }
  (junction 2) -> (junction 6) [order 1] : [tPredictTime < tMaxPredTime]
  (junction 30) -> (junction 2) [order 2]
  (junction 2) -> (junction 13) [order 2]
  (junction 6) -> (junction 8) : {
      | 	tPredictTimeOut = tPredictTime;
      | 	CollisionProbFunc;
      | }
  (junction 8) -> (junction 30) : {
      | 	tPredictTime += stepTime;
      | }
  (junction 30) -> (junction 22) [order 1] : [preCollisionProb == true]
  (junction 22) -> (junction 13) : {
      |   TTC = tPredictTimeOut;
      | }
  (junction 13) -> (junction 25)
  data: stepTime input single; tMaxPredTime input single; tPredictTimeOut output single; tPredictTime local single; preCollisionProb input; TTC output single
  events: CollisionProbFunc output FUNCTION_CALL_EVENT

== stateflow chart TosAeb/FcwAebJa_Step_sys/ALG_AEBSystem/ALG_AEBController/AEB_LatObjInfoCalcApp/LatPedObjCalc/LatPedObjCalc/latPedObjInfoCalc/ObjCrossFlgCal/PedPreCollisionCalcFunc/ObjLenthWidthCalc/ObjLengthWidthCalc ==
  (default) -> (junction 32)
  (junction 32) -> (junction 34) [order 1] : [ObjectTypeCameraConfirmed == true]
  (junction 32) -> (junction 30) [order 2] : {
      | 	ObjectLength = P_LatPedCA_PreCollisionPedLengthThres;
      | 	ObjectWidth = P_LatPedCA_PreCollisionPedWidthThres;
      | }
  (junction 34) -> (junction 36) : {
      | 	ObjectLength = ObjectLengthIn;
      | 	ObjectWidth = ObjectWidthIn;
      | }
  (junction 36) -> (junction 30)
  (junction 30) -> (junction 10)
  (junction 10) -> (junction 12) [order 1] : [(abs(ObjectYawAngle) > 45 &&...
      | abs(ObjectYawAngle) < 135)||...
      | ObjectMovingOrientation == AEB_ObjectMovingOrientation.Crossing]
  (junction 10) -> (junction 16) [order 2]
  (junction 12) -> (junction 14) : {
      |       temp = ObjectLength;
      | 	ObjectLength = ObjectWidth;
      | 	ObjectWidth = temp;
      | }
  (junction 14) -> (junction 16)
  (junction 16) -> (junction 19) : {
      |    ObjectLength = min(ObjectLength,1.5);
      |    ObjectWidth = min(ObjectWidth,1.5);
      | }
  data: ObjectYawAngle input; ObjectMovingOrientation input; ObjectTypeCameraConfirmed input; ObjectLengthIn input; ObjectWidthIn input; ObjectLength output single; ObjectWidth output single; P_LatPedCA_PreCollisionPedLengthThres input; P_LatPedCA_PreCollisionPedWidthThres input; temp local single

== stateflow chart TosAeb/FcwAebJa_Step_sys/ALG_AEBSystem/ALG_AEBController/AEB_LatObjInfoCalcApp/LatPedObjCalc/Subsystem/latPedObjInfoCalc/ObjColiisionRiskProbCalc/CollisionRiskProbCalc/CollisionRiskProbCalc/dxOverlapFlow ==
  (default) -> (junction 32) : {
      | 	dxOverlapLimit = dxReginObjRUCorr - dxReginObjLLCorr;
      | 	dxOverlapLimit = max(dxOverlapLimit,0.1);
      | }
  (junction 32) -> (junction 34) [order 1] : [dxReginObjLLCorr > dxReginEgoRUCorr || ...
      | dxReginObjRUCorr < dxReginEgoLLCorr]
  (junction 32) -> (junction 2) [order 2]
  (junction 34) -> (junction 36) : {
      | 	dxOverlap = 0;
      | }
  (junction 2) -> (junction 3) [order 1] : [dxReginEgoRUCorr - dxReginEgoLLCorr < dxOverlapLimit && ...
      | dxReginEgoLLCorr >= dxReginObjLLCorr &&...
      | dxReginEgoRUCorr < dxReginObjRUCorr]
  (junction 2) -> (junction 7) [order 2]
  (junction 3) -> (junction 5) : {
      | 	dxOverlap = dxReginEgoRUCorr - dxReginEgoLLCorr;
      | }
  (junction 7) -> (junction 9) [order 1] : [dxReginEgoRUCorr - dxReginObjLLCorr < dxOverlapLimit &&...
      | dxReginEgoRUCorr - dxReginObjLLCorr > 0 && ...
      | dxReginEgoLLCorr < dxReginObjLLCorr]
  (junction 7) -> (junction 13) [order 2]
  (junction 9) -> (junction 11) : {
      | 	dxOverlap = dxReginEgoRUCorr - dxReginObjLLCorr;
      | }
  (junction 13) -> (junction 15) [order 1] : [dxReginObjRUCorr - dxReginEgoLLCorr< dxOverlapLimit && ...
      | dxReginObjRUCorr - dxReginEgoLLCorr> 0 && ...
      | dxReginEgoRUCorr > dxReginObjRUCorr]
  (junction 13) -> (junction 19) [order 2] : {
      | 	dxOverlap= dxOverlapLimit;
      | }
  (junction 15) -> (junction 17) : {
      | 	dxOverlap = dxReginObjRUCorr - dxReginEgoLLCorr;
      | }
  (junction 17) -> (junction 19)
  (junction 11) -> (junction 17)
  (junction 5) -> (junction 11)
  (junction 36) -> (junction 5)
  (junction 19) -> (junction 22)
  data: dxReginEgoRUCorr input single; dxReginEgoLLCorr input single; dxReginObjRUCorr input single; dxReginObjLLCorr input single; dxOverlap output single; dxOverlapLimit output single

== stateflow chart TosAeb/FcwAebJa_Step_sys/ALG_AEBSystem/ALG_AEBController/AEB_LatObjInfoCalcApp/LatPedObjCalc/LatPedObjCalc/latPedObjInfoCalc/ObjCrossFlgCal/PedPreCollisionCalcFunc/PreCrossFlgCalcFlow ==
  state pedPreCollisionFlg = VectorCrossAlgorithm(x1,y1,x2,y2,m1,n1,m2,n2) [function]
  (default) -> (junction 59) : {
      | 	pedPreCollisionFlg = false;
      | 	i = 0;
      | }
  (junction 59) -> (junction 58) [order 1] : [ i < 4]
  (junction 58) -> (junction 48) : {
      | 	p = (i+1)%%4;
      | 	j = 0;
      | }
  (junction 48) -> (junction 46) [order 1] : [j < 4]
  (junction 46) -> (junction 49) : {
      | 	q = (j+1)%%4;
      | 	pedPreCollisionFlg = VectorCrossAlgorithm(subjDxPre[i],subjDyPre[i],subjDxPre[p],subjDyPre[p],...
      | 	objDxPre[j],objDyPre[j],objDxPre[q],objDyPre[q]);
      | }
  (junction 60) -> (junction 59) : {
      | 	i++;
      | }
  (junction 59) -> (junction 61) [order 2]
  (junction 49) -> (junction 48) [order 2] : {
      | 	j++;
      | }
  (junction 48) -> (junction 60) [order 2]
  (junction 49) -> (junction 64) [order 1] : [pedPreCollisionFlg == true]
  (junction 64) -> (junction 61)
  (junction 61) -> (junction 67)
  data: x1 function_input single; y1 function_input single; x2 function_input single; y2 function_input single; m1 function_input single; n1 function_input single; m2 function_input single; n2 function_input single; pedPreCollisionFlg function_output; subjDxPre input single; subjDyPre input single; objDxPre input single; objDyPre input single; j local single; i local single; p local single; q local single; pedPreCollisionFlg output boolean

== stateflow chart TosAeb/FcwAebJa_Step_sys/ALG_AEBSystem/ALG_AEBController/AEB_LatObjInfoCalcApp/LatPedObjCalc/TargetSelectCalc/tgtObjFlow1 ==
  state TargetSelectFunc [function]
    (default) -> (junction 63)
    (junction 63) -> (junction 75) [order 1] : [ObjPreSelectCondFufilled(index) == false]
    (junction 63) -> (junction 213) [order 2] : {
        | 	tgtIsFind = true;
        |
        | }
    (junction 75) -> (junction 72)
    (junction 213) -> (junction 215) [order 1] : [ObjPreSelectCondFufilled(tgtIndex) == false]
    (junction 213) -> (junction 94) [order 2]
    (junction 215) -> (junction 218) : {
        | 	tgtIndex = index;
        | }
    (junction 94) -> (junction 93) [order 1] : [latPedCalcInfo(index).CollisionRiskProb > P_LatPedCA_SelectObjCollisionRiskProbThres]
    (junction 94) -> (junction 142) [order 2]
    (junction 93) -> (junction 210)
    (junction 210) -> (junction 128) : {
        | 	temp1=abs(latPedCalcInfo(index).latPedObjRawInfo.dy);
        | 	temp2 = abs(latPedCalcInfo(tgtIndex).latPedObjRawInfo.dy);
        | }
    (junction 128) -> (junction 131) [order 1] : [temp1 < temp2]
    (junction 128) -> (junction 129) [order 2] : {
        | 		temp3 = temp1 - temp2;
        | }
    (junction 131) -> (junction 133) : {
        | 	tgtIndex = index;
        | }
    (junction 142) -> (junction 143) [order 1] : [latPedCalcInfo(index).CollisionRiskProb > ...
        | latPedCalcInfo(tgtIndex).CollisionRiskProb]
    (junction 142) -> (junction 149) [order 2] : {
        | 	temp1_c =abs(latPedCalcInfo(index).CollisionRiskProb);
        | 	temp2_c = abs(latPedCalcInfo(tgtIndex).CollisionRiskProb);
        | 	temp3_c = temp1_c - temp2_c;
        |
        | }
    (junction 143) -> (junction 145) : {
        | 	tgtIndex = index;
        | }
    (junction 129) -> (junction 137) [order 1] : [abs(temp3) < 1e-6]
    (junction 129) -> (junction 101) [order 2]
    (junction 137) -> (junction 56)
    (junction 56) -> (junction 103) [order 1] : [latPedCalcInfo(index).CollisionRiskProb > ...
        | latPedCalcInfo(tgtIndex).CollisionRiskProb]
    (junction 56) -> (junction 88) [order 2]
    (junction 103) -> (junction 59) : {
        | 	tgtIndex = index;
        | }
    (junction 149) -> (junction 151) [order 1] : [abs(temp3_c) < 1e-6]
    (junction 149) -> (junction 69) [order 2]
    (junction 151) -> (junction 157) : {
        | 	temp1=abs(latPedCalcInfo(index).latPedObjRawInfo.dy);
        | 	temp2 = abs(latPedCalcInfo(tgtIndex).latPedObjRawInfo.dy);
        | }
    (junction 157) -> (junction 158) [order 1] : [temp1 < temp2]
    (junction 157) -> (junction 153) [order 2]
    (junction 158) -> (junction 160) : {
        | 	tgtIndex = index;
        | }
    (junction 153) -> (junction 69)
    (junction 160) -> (junction 153)
    (junction 145) -> (junction 160)
    (junction 101) -> (junction 145)
    (junction 88) -> (junction 101)
    (junction 59) -> (junction 88)
    (junction 133) -> (junction 59)
    (junction 218) -> (junction 133)
    (junction 72) -> (junction 218)
    (junction 69) -> (junction 81)
  state TgtIndexSearch [function]
    (default) -> (junction 226)
    (junction 226) -> (junction 227) : {
        |     index = 1;
        | }
    (junction 227) -> (junction 228) [order 1] : [index <= 10]
    (junction 231) -> (junction 227)
    (junction 227) -> (junction 232) [order 2]
    (junction 228) -> (junction 229)
    (junction 229) -> (junction 230) [order 1] : [latPedCalcInfo(index).latPedObjRawInfo.ObjectID == tgtIndexK1]
    (junction 229) -> (junction 231) [order 2] : {
        |    index = index+1;
        | }
    (junction 230) -> (junction 233) : {
        |    tgtIndexK1 = index;
        | }
    (junction 233) -> (junction 232)
    (junction 232) -> (junction 234)
  (default) -> (junction 195) : {
      | 	tgtIndex = 1;
      | 	tgtIsFind = false;
      |       tgtIndexK1 = uint8(1);
      | }
  (junction 195) -> (junction 184) [order 1] : [TargetIDK1 ~= CNobjInvalidID]
  (junction 195) -> (junction 111) [order 2]
  (junction 184) -> (junction 197) : {
      |    TgtIndexSearch();
      | }
  (junction 197) -> (junction 199) [order 1] : [ObjPreSelectCondFufilled(tgtIndexK1) == true]
  (junction 197) -> (junction 201) [order 2]
  (junction 199) -> (junction 185)
  (junction 201) -> (junction 111)
  (junction 185) -> (junction 201) [order 2]
  (junction 185) -> (junction 183) [order 1] : [latPedCalcInfo(tgtIndexK1).CollisionRiskProb >P_LatPedCA_SelectTgtCollisionRiskProbThres ]
  (junction 111) -> (junction 112) : {
      | 	index = uint8(1);
      | }
  (junction 183) -> (junction 205) : {
      | 	tgtIndex = tgtIndexK1;
      | 	tgtIsFind = true;
      | }
  (junction 112) -> (junction 110) [order 1] : [index <= 10]
  (junction 169) -> (junction 112)
  (junction 112) -> (junction 206) [order 2]
  (junction 110) -> (junction 113) : {
      | 	TargetSelectFunc();
      | }
  (junction 113) -> (junction 169) : {
      | 	index = index + 1;
      | }
  (junction 205) -> (junction 206)
  (junction 206) -> (junction 114)
  (junction 114) -> (junction 172) [order 1] : [tgtIsFind == true]
  (junction 114) -> (junction 176) [order 2] : {
      | 	tgtObj = latPedObjCalcInfoDefaultParams;
      | }
  (junction 172) -> (junction 174) : {
      | 	tgtObj = latPedCalcInfo(tgtIndex);
      | }
  (junction 174) -> (junction 176)
  (junction 176) -> (junction 179)
  data: latPedCalcInfo input Bus: BusLatPedObjCalcInfo size=10; ObjPreSelectCondFufilled input size=10; index local uint8; tgtObj output Bus: BusLatPedObjCalcInfo; tgtIndex output uint8; temp1 local; temp2 local; temp3 local; temp1_c local; temp2_c local; temp3_c local; tgtIsFind output; latPedObjCalcInfoDefaultParams parameter Bus: BusLatPedObjCalcInfo; CNobjInvalidID parameter; P_LatPedCA_SelectObjCollisionRiskProbThres input; P_LatPedCA_SelectTgtCollisionRiskProbThres input; TargetIDK1 input uint16; tgtIndexK1 local

== matlab function TosAeb/ELK_Objsel/MATLAB Function ==
  data: TA_Left_Flag_Vec input; TA_Right_Flag_Vec input; dx_Obj_vector input; Index_Left_Obj output; Index_Right_Obj output
function [Index_Left_Obj,Index_Right_Obj]= fcnFindTargetELK(TA_Left_Flag_Vec,TA_Right_Flag_Vec,dx_Obj_vector)

Index_Left_Obj=255;
Index_Right_Obj=255;
dxMin_Left=single(1000);
dxMin_Right=single(1000);
for i=1:32
    if( (TA_Left_Flag_Vec(i)>0) && (dx_Obj_vector(i)<dxMin_Left) )
        Index_Left_Obj=i;
        dxMin_Left=dx_Obj_vector(i);
    end
    if( (TA_Right_Flag_Vec(i)>0) && (dx_Obj_vector(i)<dxMin_Right) )
        Index_Right_Obj=i;
        dxMin_Right=dx_Obj_vector(i);
    end
end

== stateflow chart TosAeb/ELK_Objsel/Target_Selection/If Action Subsystem/ELK_TargetSelection/If Action Threat_Assessment_Left/Delay_Trig/Chart ==
  state OFF
    en: Delay_Trig =0;
    count= 0;
    settings: description=_GWMH9P4.LKAFN.111_17_
  state ON
    en: Delay_Trig = 0;
    count++;
    du:count++;
    settings: description=_GWMH9P4.LKAFN.111_18_
  state Trig
    en: Delay_Trig = 1;
    settings: description=_GWMH9P4.LKAFN.111_19_
  (default) -> OFF
  OFF -> ON : [(Input)]
  ON -> OFF [order 2] : [!Input]
  Trig -> OFF : [!Input]
  ON -> Trig [order 1] : [(Input) && (count>= Delay_Trig_count)]
  data: Input input; Delay_Trig_count input; Delay_Trig output double; count local double

== stateflow chart TosAeb/ELK_Objsel/Target_Selection/If Action Subsystem/ELK_TargetSelection/If Action Threat_Assessment_Left/Delay_Trig1/Chart ==
  state OFF
    en: Delay_Trig =0;
    count= 0;
    settings: description=_GWMH9P4.LKAFN.111_17_
  state ON
    en: Delay_Trig = 0;
    count++;
    du:count++;
    settings: description=_GWMH9P4.LKAFN.111_18_
  state Trig
    en: Delay_Trig = 1;
    settings: description=_GWMH9P4.LKAFN.111_19_
  (default) -> OFF
  OFF -> ON : [(Input)]
  ON -> OFF [order 2] : [!Input]
  Trig -> OFF : [!Input]
  ON -> Trig [order 1] : [(Input) && (count>= Delay_Trig_count)]
  data: Input input; Delay_Trig_count input; Delay_Trig output double; count local double

== stateflow chart TosAeb/ELK_Objsel/Target_Selection/If Action Subsystem/ELK_TargetSelection/If Action Threat_Assessment_Left/Hysteresis ==
  state Flag_False
    en:
    Flag = 0;
    du:
    Flag = 0;
    settings: description=_GWMH9P5.ELKFN.111_3_
  state Flag_True
    en:
    Flag = 1;
    du:
    Flag = 1;
    settings: description=_GWMH9P5.ELKFN.111_4_
  (default) -> Flag_False
  Flag_False -> Flag_True : [Input_1 == 1]
  Flag_True -> Flag_False : [Input_2 == 1]
  data: Input_1 input; Input_2 input; Flag output double

== stateflow chart TosAeb/ELK_Objsel/Target_Selection/If Action Subsystem/ELK_TargetSelection/If Action Threat_Assessment_Left/Hysteresis1 ==
  state Flag_False
    en:
    Flag = 0;
    du:
    Flag = 0;
    settings: description=_GWMH9P5.ELKFN.111_3_
  state Flag_True
    en:
    Flag = 1;
    du:
    Flag = 1;
    settings: description=_GWMH9P5.ELKFN.111_4_
  (default) -> Flag_False
  Flag_False -> Flag_True : [Input_1 == 1]
  Flag_True -> Flag_False : [Input_2 == 1]
  data: Input_1 input; Input_2 input; Flag output double

== stateflow chart TosAeb/ELK_Objsel/Target_Selection/If Action Subsystem/ELK_TargetSelection/If Action Threat_Assessment_Left/Hysteresis2 ==
  state Flag_False
    en:
    Flag = 0;
    du:
    Flag = 0;
    settings: description=_GWMH9P5.ELKFN.111_3_
  state Flag_True
    en:
    Flag = 1;
    du:
    Flag = 1;
    settings: description=_GWMH9P5.ELKFN.111_4_
  (default) -> Flag_False
  Flag_False -> Flag_True : [Input_1 == 1]
  Flag_True -> Flag_False : [Input_2 == 1]
  data: Input_1 input; Input_2 input; Flag output double

== stateflow chart TosAeb/ELK_Objsel/Target_Selection/If Action Subsystem/ELK_TargetSelection/If Action Threat_Assessment_Left/Trigger Hold/Chart ==
  (default) -> (junction 1)
  (junction 1) -> (junction 2) [order 1] : [(Trigger==1)]...
      | {count = 0;}
  (junction 1) -> (junction 12) [order 2]
  (junction 2) -> (junction 10)
  (junction 12) -> (junction 10) [order 1] : [(count<= hold_time)&&(count>0)]
  (junction 12) -> (junction 3) [order 2] : {Out = 0;}
  (junction 10) -> (junction 15) : {
      | Out = 1;...
      | count = count + 10;
      | }
  (junction 3) -> (junction 15)
  (junction 15) -> (junction 8)
  data: Trigger input; hold_time input; Out output double; count local double
